simulink model "os_polyphase_synth_4096c"  (R2021a) (continued)
== system os_polyphase_synth_4096c/pfb_fir/pol1_in1_tap13 == (continued)
      | P>>17-(ACIN+D)*BCIN-C-CARRYIN
      | P>>17-(ACIN+D)*BCIN-CARRYIN
      | P>>17-(ACIN+D)*BCIN-P
      | P>>17-(ACIN+D)*BCIN-P-CARRYIN
      | P>>17-(ACIN+D)-C
      | P>>17-(ACIN+D)-C-CARRYIN
      | P>>17-(ACIN+D)-CARRYIN
      | P>>17-(ACIN+D)-P
      | P>>17-(ACIN+D)-P-CARRYIN
      | P>>17-(ACIN+P)
      | P>>17-(ACIN+P+CARRYIN)
      | P>>17-(B*(A+D))
      | P>>17-(B*(A+D)+C)
      | P>>17-(B*(A+D)+C+CARRYIN)
      | P>>17-(B*(A+D)+CARRYIN)
      | P>>17-(B*(A+D)+P)
      | P>>17-(B*(A+D)+P+CARRYIN)
      | P>>17-(B*(ACIN+D))
      | P>>17-(B*(ACIN+D)+C)
      | P>>17-(B*(ACIN+D)+C+CARRYIN)
      | P>>17-(B*(ACIN+D)+CARRYIN)
      | P>>17-(B*(ACIN+D)+P)
      | P>>17-(B*(ACIN+D)+P+CARRYIN)
      | P>>17-(B*(D+A))
      | P>>17-(B*(D+A)+C)
      | P>>17-(B*(D+A)+C+CARRYIN)
      | P>>17-(B*(D+A)+CARRYIN)
      | P>>17-(B*(D+A)+P)
      | P>>17-(B*(D+A)+P+CARRYIN)
      | P>>17-(B*(D+ACIN))
      | P>>17-(B*(D+ACIN)+C)
      | P>>17-(B*(D+ACIN)+C+CARRYIN)
      | P>>17-(B*(D+ACIN)+CARRYIN)
      | P>>17-(B*(D+ACIN)+P)
      | P>>17-(B*(D+ACIN)+P+CARRYIN)
      | P>>17-(B*(D-A))
      | P>>17-(B*(D-A)+C)
      | P>>17-(B*(D-A)+C+CARRYIN)
      | P>>17-(B*(D-A)+CARRYIN)
      | P>>17-(B*(D-A)+P)
      | P>>17-(B*(D-A)+P+CARRYIN)
      | P>>17-(B*(D-ACIN))
      | P>>17-(B*(D-ACIN)+C)
      | P>>17-(B*(D-ACIN)+C+CARRYIN)
      | P>>17-(B*(D-ACIN)+CARRYIN)
      | P>>17-(B*(D-ACIN)+P)
      | P>>17-(B*(D-ACIN)+P+CARRYIN)
      | P>>17-(B*A)
      | P>>17-(B*A+C)
      | P>>17-(B*A+C+CARRYIN)
      | P>>17-(B*A+CARRYIN)
      | P>>17-(B*A+P)
      | P>>17-(B*A+P+CARRYIN)
      | P>>17-(B*ACIN)
      | P>>17-(B*ACIN+C)
      | P>>17-(B*ACIN+C+CARRYIN)
      | P>>17-(B*ACIN+CARRYIN)
      | P>>17-(B*ACIN+P)
      | P>>17-(B*ACIN+P+CARRYIN)
      | P>>17-(B*D)
      | P>>17-(B*D+C)
      | P>>17-(B*D+C+CARRYIN)
      | P>>17-(B*D+CARRYIN)
      | P>>17-(B*D+P)
      | P>>17-(B*D+P+CARRYIN)
      | P>>17-(BCIN*(A+D))
      | P>>17-(BCIN*(A+D)+C)
      | P>>17-(BCIN*(A+D)+C+CARRYIN)
      | P>>17-(BCIN*(A+D)+CARRYIN)
      | P>>17-(BCIN*(A+D)+P)
      | P>>17-(BCIN*(A+D)+P+CARRYIN)
      | P>>17-(BCIN*(ACIN+D))
      | P>>17-(BCIN*(ACIN+D)+C)
      | P>>17-(BCIN*(ACIN+D)+C+CARRYIN)
      | P>>17-(BCIN*(ACIN+D)+CARRYIN)
      | P>>17-(BCIN*(ACIN+D)+P)
      | P>>17-(BCIN*(ACIN+D)+P+CARRYIN)
      | P>>17-(BCIN*(D+A))
      | P>>17-(BCIN*(D+A)+C)
      | P>>17-(BCIN*(D+A)+C+CARRYIN)
      | P>>17-(BCIN*(D+A)+CARRYIN)
      | P>>17-(BCIN*(D+A)+P)
      | P>>17-(BCIN*(D+A)+P+CARRYIN)
      | P>>17-(BCIN*(D+ACIN))
      | P>>17-(BCIN*(D+ACIN)+C)
      | P>>17-(BCIN*(D+ACIN)+C+CARRYIN)
      | P>>17-(BCIN*(D+ACIN)+CARRYIN)
      | P>>17-(BCIN*(D+ACIN)+P)
      | P>>17-(BCIN*(D+ACIN)+P+CARRYIN)
      | P>>17-(BCIN*(D-A))
      | P>>17-(BCIN*(D-A)+C)
      | P>>17-(BCIN*(D-A)+C+CARRYIN)
      | P>>17-(BCIN*(D-A)+CARRYIN)
      | P>>17-(BCIN*(D-A)+P)
      | P>>17-(BCIN*(D-A)+P+CARRYIN)
      | P>>17-(BCIN*(D-ACIN))
      | P>>17-(BCIN*(D-ACIN)+C)
      | P>>17-(BCIN*(D-ACIN)+C+CARRYIN)
      | P>>17-(BCIN*(D-ACIN)+CARRYIN)
      | P>>17-(BCIN*(D-ACIN)+P)
      | P>>17-(BCIN*(D-ACIN)+P+CARRYIN)
      | P>>17-(BCIN*A)
      | P>>17-(BCIN*A+C)
      | P>>17-(BCIN*A+C+CARRYIN)
      | P>>17-(BCIN*A+CARRYIN)
      | P>>17-(BCIN*A+P)
      | P>>17-(BCIN*A+P+CARRYIN)
      | P>>17-(BCIN*ACIN)
      | P>>17-(BCIN*ACIN+C)
      | P>>17-(BCIN*ACIN+C+CARRYIN)
      | P>>17-(BCIN*ACIN+CARRYIN)
      | P>>17-(BCIN*ACIN+P)
      | P>>17-(BCIN*ACIN+P+CARRYIN)
      | P>>17-(BCIN*D)
      | P>>17-(BCIN*D+C)
      | P>>17-(BCIN*D+C+CARRYIN)
      | P>>17-(BCIN*D+CARRYIN)
      | P>>17-(BCIN*D+P)
      | P>>17-(BCIN*D+P+CARRYIN)
      | P>>17-(C)
      | P>>17-(C+(A+D))
      | P>>17-(C+(A+D)*(A+D))
      | P>>17-(C+(A+D)*(A+D)+CARRYIN)
      | P>>17-(C+(A+D)*B)
      | P>>17-(C+(A+D)*B+CARRYIN)
      | P>>17-(C+(A+D)*BCIN)
      | P>>17-(C+(A+D)*BCIN+CARRYIN)
      | P>>17-(C+(A+D)+CARRYIN)
      | P>>17-(C+(ACIN+D))
      | P>>17-(C+(ACIN+D)*(ACIN+D))
      | P>>17-(C+(ACIN+D)*(ACIN+D)+CARRYIN)
      | P>>17-(C+(ACIN+D)*B)
      | P>>17-(C+(ACIN+D)*B+CARRYIN)
      | P>>17-(C+(ACIN+D)*BCIN)
      | P>>17-(C+(ACIN+D)*BCIN+CARRYIN)
      | P>>17-(C+(ACIN+D)+CARRYIN)
      | P>>17-(C+(D+A))
      | P>>17-(C+(D+A)*(D+A))
      | P>>17-(C+(D+A)*(D+A)+CARRYIN)
      | P>>17-(C+(D+A)*B)
      | P>>17-(C+(D+A)*B+CARRYIN)
      | P>>17-(C+(D+A)*BCIN)
      | P>>17-(C+(D+A)*BCIN+CARRYIN)
      | P>>17-(C+(D+A)+CARRYIN)
      | P>>17-(C+(D+ACIN))
      | P>>17-(C+(D+ACIN)*(D+ACIN))
      | P>>17-(C+(D+ACIN)*(D+ACIN)+CARRYIN)
      | P>>17-(C+(D+ACIN)*B)
      | P>>17-(C+(D+ACIN)*B+CARRYIN)
      | P>>17-(C+(D+ACIN)*BCIN)
      | P>>17-(C+(D+ACIN)*BCIN+CARRYIN)
      | P>>17-(C+(D+ACIN)+CARRYIN)
      | P>>17-(C+(D-A))
      | P>>17-(C+(D-A)*(D-A))
      | P>>17-(C+(D-A)*(D-A)+CARRYIN)
      | P>>17-(C+(D-A)*B)
      | P>>17-(C+(D-A)*B+CARRYIN)
      | P>>17-(C+(D-A)*BCIN)
      | P>>17-(C+(D-A)*BCIN+CARRYIN)
      | P>>17-(C+(D-A)+CARRYIN)
      | P>>17-(C+(D-ACIN))
      | P>>17-(C+(D-ACIN)*(D-ACIN))
      | P>>17-(C+(D-ACIN)*(D-ACIN)+CARRYIN)
      | P>>17-(C+(D-ACIN)*B)
      | P>>17-(C+(D-ACIN)*B+CARRYIN)
      | P>>17-(C+(D-ACIN)*BCIN)
      | P>>17-(C+(D-ACIN)*BCIN+CARRYIN)
      | P>>17-(C+(D-ACIN)+CARRYIN)
      | P>>17-(C+A)
      | P>>17-(C+A*A)
      | P>>17-(C+A*A+CARRYIN)
      | P>>17-(C+A*B)
      | P>>17-(C+A*B+CARRYIN)
      | P>>17-(C+A*BCIN)
      | P>>17-(C+A*BCIN+CARRYIN)
      | P>>17-(C+A+CARRYIN)
      | P>>17-(C+ACIN)
      | P>>17-(C+ACIN*ACIN)
      | P>>17-(C+ACIN*ACIN+CARRYIN)
      | P>>17-(C+ACIN*B)
      | P>>17-(C+ACIN*B+CARRYIN)
      | P>>17-(C+ACIN*BCIN)
      | P>>17-(C+ACIN*BCIN+CARRYIN)
      | P>>17-(C+ACIN+CARRYIN)
      | P>>17-(C+B*(A+D))
      | P>>17-(C+B*(A+D)+CARRYIN)
      | P>>17-(C+B*(ACIN+D))
      | P>>17-(C+B*(ACIN+D)+CARRYIN)
      | P>>17-(C+B*(D+A))
      | P>>17-(C+B*(D+A)+CARRYIN)
      | P>>17-(C+B*(D+ACIN))
      | P>>17-(C+B*(D+ACIN)+CARRYIN)
      | P>>17-(C+B*(D-A))
      | P>>17-(C+B*(D-A)+CARRYIN)
      | P>>17-(C+B*(D-ACIN))
      | P>>17-(C+B*(D-ACIN)+CARRYIN)
      | P>>17-(C+B*A)
      | P>>17-(C+B*A+CARRYIN)
      | P>>17-(C+B*ACIN)
      | P>>17-(C+B*ACIN+CARRYIN)
      | P>>17-(C+B*D)
      | P>>17-(C+B*D+CARRYIN)
      | P>>17-(C+BCIN*(A+D))
      | P>>17-(C+BCIN*(A+D)+CARRYIN)
      | P>>17-(C+BCIN*(ACIN+D))
      | P>>17-(C+BCIN*(ACIN+D)+CARRYIN)
      | P>>17-(C+BCIN*(D+A))
      | P>>17-(C+BCIN*(D+A)+CARRYIN)
      | P>>17-(C+BCIN*(D+ACIN))
      | P>>17-(C+BCIN*(D+ACIN)+CARRYIN)
      | P>>17-(C+BCIN*(D-A))
      | P>>17-(C+BCIN*(D-A)+CARRYIN)
      | P>>17-(C+BCIN*(D-ACIN))
      | P>>17-(C+BCIN*(D-ACIN)+CARRYIN)
      | P>>17-(C+BCIN*A)
      | P>>17-(C+BCIN*A+CARRYIN)
      | P>>17-(C+BCIN*ACIN)
      | P>>17-(C+BCIN*ACIN+CARRYIN)
      | P>>17-(C+BCIN*D)
      | P>>17-(C+BCIN*D+CARRYIN)
      | P>>17-(C+C)
      | P>>17-(C+C+CARRYIN)
      | P>>17-(C+C+CONCAT)
      | P>>17-(C+C+CONCAT+CARRYIN)
      | P>>17-(C+C+P)
      | P>>17-(C+C+P+CARRYIN)
      | P>>17-(C+CARRYIN)
      | P>>17-(C+CONCAT)
      | P>>17-(C+CONCAT+C)
      | P>>17-(C+CONCAT+C+CARRYIN)
      | P>>17-(C+CONCAT+CARRYIN)
      | P>>17-(C+CONCAT+P)
      | P>>17-(C+CONCAT+P+CARRYIN)
      | P>>17-(C+D)
      | P>>17-(C+D*B)
      | P>>17-(C+D*B+CARRYIN)
      | P>>17-(C+D*BCIN)
      | P>>17-(C+D*BCIN+CARRYIN)
      | P>>17-(C+D*D)
      | P>>17-(C+D*D+CARRYIN)
      | P>>17-(C+D+CARRYIN)
      | P>>17-(C+P)
      | P>>17-(C+P+C)
      | P>>17-(C+P+C+CARRYIN)
      | P>>17-(C+P+CARRYIN)
      | P>>17-(C+P+CONCAT)
      | P>>17-(C+P+CONCAT+CARRYIN)
      | P>>17-(C+P+P)
      | P>>17-(C+P+P+CARRYIN)
      | P>>17-(CARRYIN)
      | P>>17-(CONCAT)
      | P>>17-(CONCAT+C)
      | P>>17-(CONCAT+C+C)
      | P>>17-(CONCAT+C+C+CARRYIN)
      | P>>17-(CONCAT+C+CARRYIN)
      | P>>17-(CONCAT+C+P)
      | P>>17-(CONCAT+C+P+CARRYIN)
      | P>>17-(CONCAT+CARRYIN)
      | P>>17-(CONCAT+P)
      | P>>17-(CONCAT+P+C)
      | P>>17-(CONCAT+P+C+CARRYIN)
      | P>>17-(CONCAT+P+CARRYIN)
      | P>>17-(D)
      | P>>17-(D*B)
      | P>>17-(D*B+C)
      | P>>17-(D*B+C+CARRYIN)
      | P>>17-(D*B+CARRYIN)
      | P>>17-(D*B+P)
      | P>>17-(D*B+P+CARRYIN)
      | P>>17-(D*BCIN)
      | P>>17-(D*BCIN+C)
      | P>>17-(D*BCIN+C+CARRYIN)
      | P>>17-(D*BCIN+CARRYIN)
      | P>>17-(D*BCIN+P)
      | P>>17-(D*BCIN+P+CARRYIN)
      | P>>17-(D*D)
      | P>>17-(D*D+C)
      | P>>17-(D*D+C+CARRYIN)
      | P>>17-(D*D+CARRYIN)
      | P>>17-(D*D+P)
      | P>>17-(D*D+P+CARRYIN)
      | P>>17-(D+A)
      | P>>17-(D+A)*(D+A)
      | P>>17-(D+A)*(D+A)-C
      | P>>17-(D+A)*(D+A)-C-CARRYIN
      | P>>17-(D+A)*(D+A)-CARRYIN
      | P>>17-(D+A)*(D+A)-P
      | P>>17-(D+A)*(D+A)-P-CARRYIN
      | P>>17-(D+A)*B
      | P>>17-(D+A)*B-C
      | P>>17-(D+A)*B-C-CARRYIN
      | P>>17-(D+A)*B-CARRYIN
      | P>>17-(D+A)*B-P
      | P>>17-(D+A)*B-P-CARRYIN
      | P>>17-(D+A)*BCIN
      | P>>17-(D+A)*BCIN-C
      | P>>17-(D+A)*BCIN-C-CARRYIN
      | P>>17-(D+A)*BCIN-CARRYIN
      | P>>17-(D+A)*BCIN-P
      | P>>17-(D+A)*BCIN-P-CARRYIN
      | P>>17-(D+A)-C
      | P>>17-(D+A)-C-CARRYIN
      | P>>17-(D+A)-CARRYIN
      | P>>17-(D+A)-P
      | P>>17-(D+A)-P-CARRYIN
      | P>>17-(D+ACIN)
      | P>>17-(D+ACIN)*(D+ACIN)
      | P>>17-(D+ACIN)*(D+ACIN)-C
      | P>>17-(D+ACIN)*(D+ACIN)-C-CARRYIN
      | P>>17-(D+ACIN)*(D+ACIN)-CARRYIN
      | P>>17-(D+ACIN)*(D+ACIN)-P
      | P>>17-(D+ACIN)*(D+ACIN)-P-CARRYIN
      | P>>17-(D+ACIN)*B
      | P>>17-(D+ACIN)*B-C
      | P>>17-(D+ACIN)*B-C-CARRYIN
      | P>>17-(D+ACIN)*B-CARRYIN
      | P>>17-(D+ACIN)*B-P
      | P>>17-(D+ACIN)*B-P-CARRYIN
      | P>>17-(D+ACIN)*BCIN
      | P>>17-(D+ACIN)*BCIN-C
      | P>>17-(D+ACIN)*BCIN-C-CARRYIN
      | P>>17-(D+ACIN)*BCIN-CARRYIN
      | P>>17-(D+ACIN)*BCIN-P
      | P>>17-(D+ACIN)*BCIN-P-CARRYIN
      | P>>17-(D+ACIN)-C
      | P>>17-(D+ACIN)-C-CARRYIN
      | P>>17-(D+ACIN)-CARRYIN
      | P>>17-(D+ACIN)-P
      | P>>17-(D+ACIN)-P-CARRYIN
      | P>>17-(D+C)
      | P>>17-(D+C+CARRYIN)
      | P>>17-(D+CARRYIN)
      | P>>17-(D+P)
      | P>>17-(D+P+CARRYIN)
      | P>>17-(D-A)
      | P>>17-(D-A)*(D-A)
      | P>>17-(D-A)*(D-A)-C
      | P>>17-(D-A)*(D-A)-C-CARRYIN
      | P>>17-(D-A)*(D-A)-CARRYIN
      | P>>17-(D-A)*(D-A)-P
      | P>>17-(D-A)*(D-A)-P-CARRYIN
      | P>>17-(D-A)*B
      | P>>17-(D-A)*B-C
      | P>>17-(D-A)*B-C-CARRYIN
      | P>>17-(D-A)*B-CARRYIN
      | P>>17-(D-A)*B-P
      | P>>17-(D-A)*B-P-CARRYIN
      | P>>17-(D-A)*BCIN
      | P>>17-(D-A)*BCIN-C
      | P>>17-(D-A)*BCIN-C-CARRYIN
      | P>>17-(D-A)*BCIN-CARRYIN
      | P>>17-(D-A)*BCIN-P
      | P>>17-(D-A)*BCIN-P-CARRYIN
      | P>>17-(D-A)-C
      | P>>17-(D-A)-C-CARRYIN
      | P>>17-(D-A)-CARRYIN
      | P>>17-(D-A)-P
      | P>>17-(D-A)-P-CARRYIN
      | P>>17-(D-ACIN)
      | P>>17-(D-ACIN)*(D-ACIN)
      | P>>17-(D-ACIN)*(D-ACIN)-C
      | P>>17-(D-ACIN)*(D-ACIN)-C-CARRYIN
      | P>>17-(D-ACIN)*(D-ACIN)-CARRYIN
      | P>>17-(D-ACIN)*(D-ACIN)-P
      | P>>17-(D-ACIN)*(D-ACIN)-P-CARRYIN
      | P>>17-(D-ACIN)*B
      | P>>17-(D-ACIN)*B-C
      | P>>17-(D-ACIN)*B-C-CARRYIN
      | P>>17-(D-ACIN)*B-CARRYIN
      | P>>17-(D-ACIN)*B-P
      | P>>17-(D-ACIN)*B-P-CARRYIN
      | P>>17-(D-ACIN)*BCIN
      | P>>17-(D-ACIN)*BCIN-C
      | P>>17-(D-ACIN)*BCIN-C-CARRYIN
      | P>>17-(D-ACIN)*BCIN-CARRYIN
      | P>>17-(D-ACIN)*BCIN-P
      | P>>17-(D-ACIN)*BCIN-P-CARRYIN
      | P>>17-(D-ACIN)-C
      | P>>17-(D-ACIN)-C-CARRYIN
      | P>>17-(D-ACIN)-CARRYIN
      | P>>17-(D-ACIN)-P
      | P>>17-(D-ACIN)-P-CARRYIN
      | P>>17-(P)
      | P>>17-(P+(A+D))
      | P>>17-(P+(A+D)*(A+D))
      | P>>17-(P+(A+D)*(A+D)+CARRYIN)
      | P>>17-(P+(A+D)*B)
      | P>>17-(P+(A+D)*B+CARRYIN)
      | P>>17-(P+(A+D)*BCIN)
      | P>>17-(P+(A+D)*BCIN+CARRYIN)
      | P>>17-(P+(A+D)+CARRYIN)
      | P>>17-(P+(ACIN+D))
      | P>>17-(P+(ACIN+D)*(ACIN+D))
      | P>>17-(P+(ACIN+D)*(ACIN+D)+CARRYIN)
      | P>>17-(P+(ACIN+D)*B)
      | P>>17-(P+(ACIN+D)*B+CARRYIN)
      | P>>17-(P+(ACIN+D)*BCIN)
      | P>>17-(P+(ACIN+D)*BCIN+CARRYIN)
      | P>>17-(P+(ACIN+D)+CARRYIN)
      | P>>17-(P+(D+A))
      | P>>17-(P+(D+A)*(D+A))
      | P>>17-(P+(D+A)*(D+A)+CARRYIN)
      | P>>17-(P+(D+A)*B)
      | P>>17-(P+(D+A)*B+CARRYIN)
      | P>>17-(P+(D+A)*BCIN)
      | P>>17-(P+(D+A)*BCIN+CARRYIN)
      | P>>17-(P+(D+A)+CARRYIN)
      | P>>17-(P+(D+ACIN))
      | P>>17-(P+(D+ACIN)*(D+ACIN))
      | P>>17-(P+(D+ACIN)*(D+ACIN)+CARRYIN)
      | P>>17-(P+(D+ACIN)*B)
      | P>>17-(P+(D+ACIN)*B+CARRYIN)
      | P>>17-(P+(D+ACIN)*BCIN)
      | P>>17-(P+(D+ACIN)*BCIN+CARRYIN)
      | P>>17-(P+(D+ACIN)+CARRYIN)
      | P>>17-(P+(D-A))
      | P>>17-(P+(D-A)*(D-A))
      | P>>17-(P+(D-A)*(D-A)+CARRYIN)
      | P>>17-(P+(D-A)*B)
      | P>>17-(P+(D-A)*B+CARRYIN)
      | P>>17-(P+(D-A)*BCIN)
      | P>>17-(P+(D-A)*BCIN+CARRYIN)
      | P>>17-(P+(D-A)+CARRYIN)
      | P>>17-(P+(D-ACIN))
      | P>>17-(P+(D-ACIN)*(D-ACIN))
      | P>>17-(P+(D-ACIN)*(D-ACIN)+CARRYIN)
      | P>>17-(P+(D-ACIN)*B)
      | P>>17-(P+(D-ACIN)*B+CARRYIN)
      | P>>17-(P+(D-ACIN)*BCIN)
      | P>>17-(P+(D-ACIN)*BCIN+CARRYIN)
      | P>>17-(P+(D-ACIN)+CARRYIN)
      | P>>17-(P+A)
      | P>>17-(P+A*A)
      | P>>17-(P+A*A+CARRYIN)
      | P>>17-(P+A*B)
      | P>>17-(P+A*B+CARRYIN)
      | P>>17-(P+A*BCIN)
      | P>>17-(P+A*BCIN+CARRYIN)
      | P>>17-(P+A+CARRYIN)
      | P>>17-(P+ACIN)
      | P>>17-(P+ACIN*ACIN)
      | P>>17-(P+ACIN*ACIN+CARRYIN)
      | P>>17-(P+ACIN*B)
      | P>>17-(P+ACIN*B+CARRYIN)
      | P>>17-(P+ACIN*BCIN)
      | P>>17-(P+ACIN*BCIN+CARRYIN)
      | P>>17-(P+ACIN+CARRYIN)
      | P>>17-(P+B*(A+D))
      | P>>17-(P+B*(A+D)+CARRYIN)
      | P>>17-(P+B*(ACIN+D))
      | P>>17-(P+B*(ACIN+D)+CARRYIN)
      | P>>17-(P+B*(D+A))
      | P>>17-(P+B*(D+A)+CARRYIN)
      | P>>17-(P+B*(D+ACIN))
      | P>>17-(P+B*(D+ACIN)+CARRYIN)
      | P>>17-(P+B*(D-A))
      | P>>17-(P+B*(D-A)+CARRYIN)
      | P>>17-(P+B*(D-ACIN))
      | P>>17-(P+B*(D-ACIN)+CARRYIN)
      | P>>17-(P+B*A)
      | P>>17-(P+B*A+CARRYIN)
      | P>>17-(P+B*ACIN)
      | P>>17-(P+B*ACIN+CARRYIN)
      | P>>17-(P+B*D)
      | P>>17-(P+B*D+CARRYIN)
      | P>>17-(P+BCIN*(A+D))
      | P>>17-(P+BCIN*(A+D)+CARRYIN)
      | P>>17-(P+BCIN*(ACIN+D))
      | P>>17-(P+BCIN*(ACIN+D)+CARRYIN)
      | P>>17-(P+BCIN*(D+A))
      | P>>17-(P+BCIN*(D+A)+CARRYIN)
      | P>>17-(P+BCIN*(D+ACIN))
      | P>>17-(P+BCIN*(D+ACIN)+CARRYIN)
      | P>>17-(P+BCIN*(D-A))
      | P>>17-(P+BCIN*(D-A)+CARRYIN)
      | P>>17-(P+BCIN*(D-ACIN))
      | P>>17-(P+BCIN*(D-ACIN)+CARRYIN)
      | P>>17-(P+BCIN*A)
      | P>>17-(P+BCIN*A+CARRYIN)
      | P>>17-(P+BCIN*ACIN)
      | P>>17-(P+BCIN*ACIN+CARRYIN)
      | P>>17-(P+BCIN*D)
      | P>>17-(P+BCIN*D+CARRYIN)
      | P>>17-(P+C)
      | P>>17-(P+C+C)
      | P>>17-(P+C+C+CARRYIN)
      | P>>17-(P+C+CARRYIN)
      | P>>17-(P+C+CONCAT)
      | P>>17-(P+C+CONCAT+CARRYIN)
      | P>>17-(P+C+P)
      | P>>17-(P+C+P+CARRYIN)
      | P>>17-(P+CARRYIN)
      | P>>17-(P+CONCAT)
      | P>>17-(P+CONCAT+C)
      | P>>17-(P+CONCAT+C+CARRYIN)
      | P>>17-(P+CONCAT+CARRYIN)
      | P>>17-(P+D)
      | P>>17-(P+D*B)
      | P>>17-(P+D*B+CARRYIN)
      | P>>17-(P+D*BCIN)
      | P>>17-(P+D*BCIN+CARRYIN)
      | P>>17-(P+D*D)
      | P>>17-(P+D*D+CARRYIN)
      | P>>17-(P+D+CARRYIN)
      | P>>17-(P+P)
      | P>>17-(P+P+C)
      | P>>17-(P+P+C+CARRYIN)
      | P>>17-(P+P+CARRYIN)
      | P>>17-A
      | P>>17-A*A
      | P>>17-A*A-C
      | P>>17-A*A-C-CARRYIN
      | P>>17-A*A-CARRYIN
      | P>>17-A*A-P
      | P>>17-A*A-P-CARRYIN
      | P>>17-A*B
      | P>>17-A*B-C
      | P>>17-A*B-C-CARRYIN
      | P>>17-A*B-CARRYIN
      | P>>17-A*B-P
      | P>>17-A*B-P-CARRYIN
      | P>>17-A*BCIN
      | P>>17-A*BCIN-C
      | P>>17-A*BCIN-C-CARRYIN
      | P>>17-A*BCIN-CARRYIN
      | P>>17-A*BCIN-P
      | P>>17-A*BCIN-P-CARRYIN
      | P>>17-A-C
      | P>>17-A-C-CARRYIN
      | P>>17-A-CARRYIN
      | P>>17-A-P
      | P>>17-A-P-CARRYIN
      | P>>17-ACIN
      | P>>17-ACIN*ACIN
      | P>>17-ACIN*ACIN-C
      | P>>17-ACIN*ACIN-C-CARRYIN
      | P>>17-ACIN*ACIN-CARRYIN
      | P>>17-ACIN*ACIN-P
      | P>>17-ACIN*ACIN-P-CARRYIN
      | P>>17-ACIN*B
      | P>>17-ACIN*B-C
      | P>>17-ACIN*B-C-CARRYIN
      | P>>17-ACIN*B-CARRYIN
      | P>>17-ACIN*B-P
      | P>>17-ACIN*B-P-CARRYIN
      | P>>17-ACIN*BCIN
      | P>>17-ACIN*BCIN-C
      | P>>17-ACIN*BCIN-C-CARRYIN
      | P>>17-ACIN*BCIN-CARRYIN
      | P>>17-ACIN*BCIN-P
      | P>>17-ACIN*BCIN-P-CARRYIN
      | P>>17-ACIN-C
      | P>>17-ACIN-C-CARRYIN
      | P>>17-ACIN-CARRYIN
      | P>>17-ACIN-P
      | P>>17-ACIN-P-CARRYIN
      | P>>17-B*(A+D)
      | P>>17-B*(A+D)-C
      | P>>17-B*(A+D)-C-CARRYIN
      | P>>17-B*(A+D)-CARRYIN
      | P>>17-B*(A+D)-P
      | P>>17-B*(A+D)-P-CARRYIN
      | P>>17-B*(ACIN+D)
      | P>>17-B*(ACIN+D)-C
      | P>>17-B*(ACIN+D)-C-CARRYIN
      | P>>17-B*(ACIN+D)-CARRYIN
      | P>>17-B*(ACIN+D)-P
      | P>>17-B*(ACIN+D)-P-CARRYIN
      | P>>17-B*(D+A)
      | P>>17-B*(D+A)-C
      | P>>17-B*(D+A)-C-CARRYIN
      | P>>17-B*(D+A)-CARRYIN
      | P>>17-B*(D+A)-P
      | P>>17-B*(D+A)-P-CARRYIN
      | P>>17-B*(D+ACIN)
      | P>>17-B*(D+ACIN)-C
      | P>>17-B*(D+ACIN)-C-CARRYIN
      | P>>17-B*(D+ACIN)-CARRYIN
      | P>>17-B*(D+ACIN)-P
      | P>>17-B*(D+ACIN)-P-CARRYIN
      | P>>17-B*(D-A)
      | P>>17-B*(D-A)-C
      | P>>17-B*(D-A)-C-CARRYIN
      | P>>17-B*(D-A)-CARRYIN
      | P>>17-B*(D-A)-P
      | P>>17-B*(D-A)-P-CARRYIN
      | P>>17-B*(D-ACIN)
      | P>>17-B*(D-ACIN)-C
      | P>>17-B*(D-ACIN)-C-CARRYIN
      | P>>17-B*(D-ACIN)-CARRYIN
      | P>>17-B*(D-ACIN)-P
      | P>>17-B*(D-ACIN)-P-CARRYIN
      | P>>17-B*A
      | P>>17-B*A-C
      | P>>17-B*A-C-CARRYIN
      | P>>17-B*A-CARRYIN
      | P>>17-B*A-P
      | P>>17-B*A-P-CARRYIN
      | P>>17-B*ACIN
      | P>>17-B*ACIN-C
      | P>>17-B*ACIN-C-CARRYIN
      | P>>17-B*ACIN-CARRYIN
      | P>>17-B*ACIN-P
      | P>>17-B*ACIN-P-CARRYIN
      | P>>17-B*D
      | P>>17-B*D-C
      | P>>17-B*D-C-CARRYIN
      | P>>17-B*D-CARRYIN
      | P>>17-B*D-P
      | P>>17-B*D-P-CARRYIN
      | P>>17-BCIN*(A+D)
      | P>>17-BCIN*(A+D)-C
      | P>>17-BCIN*(A+D)-C-CARRYIN
      | P>>17-BCIN*(A+D)-CARRYIN
      | P>>17-BCIN*(A+D)-P
      | P>>17-BCIN*(A+D)-P-CARRYIN
      | P>>17-BCIN*(ACIN+D)
      | P>>17-BCIN*(ACIN+D)-C
      | P>>17-BCIN*(ACIN+D)-C-CARRYIN
      | P>>17-BCIN*(ACIN+D)-CARRYIN
      | P>>17-BCIN*(ACIN+D)-P
      | P>>17-BCIN*(ACIN+D)-P-CARRYIN
      | P>>17-BCIN*(D+A)
      | P>>17-BCIN*(D+A)-C
      | P>>17-BCIN*(D+A)-C-CARRYIN
      | P>>17-BCIN*(D+A)-CARRYIN
      | P>>17-BCIN*(D+A)-P
      | P>>17-BCIN*(D+A)-P-CARRYIN
      | P>>17-BCIN*(D+ACIN)
      | P>>17-BCIN*(D+ACIN)-C
      | P>>17-BCIN*(D+ACIN)-C-CARRYIN
      | P>>17-BCIN*(D+ACIN)-CARRYIN
      | P>>17-BCIN*(D+ACIN)-P
      | P>>17-BCIN*(D+ACIN)-P-CARRYIN
      | P>>17-BCIN*(D-A)
      | P>>17-BCIN*(D-A)-C
      | P>>17-BCIN*(D-A)-C-CARRYIN
      | P>>17-BCIN*(D-A)-CARRYIN
      | P>>17-BCIN*(D-A)-P
      | P>>17-BCIN*(D-A)-P-CARRYIN
      | P>>17-BCIN*(D-ACIN)
      | P>>17-BCIN*(D-ACIN)-C
      | P>>17-BCIN*(D-ACIN)-C-CARRYIN
      | P>>17-BCIN*(D-ACIN)-CARRYIN
      | P>>17-BCIN*(D-ACIN)-P
      | P>>17-BCIN*(D-ACIN)-P-CARRYIN
      | P>>17-BCIN*A
      | P>>17-BCIN*A-C
      | P>>17-BCIN*A-C-CARRYIN
      | P>>17-BCIN*A-CARRYIN
      | P>>17-BCIN*A-P
      | P>>17-BCIN*A-P-CARRYIN
      | P>>17-BCIN*ACIN
      | P>>17-BCIN*ACIN-C
      | P>>17-BCIN*ACIN-C-CARRYIN
      | P>>17-BCIN*ACIN-CARRYIN
      | P>>17-BCIN*ACIN-P
      | P>>17-BCIN*ACIN-P-CARRYIN
      | P>>17-BCIN*D
      | P>>17-BCIN*D-C
      | P>>17-BCIN*D-C-CARRYIN
      | P>>17-BCIN*D-CARRYIN
      | P>>17-BCIN*D-P
      | P>>17-BCIN*D-P-CARRYIN
      | P>>17-C
      | P>>17-C-(A+D)
      | P>>17-C-(A+D)*(A+D)
      | P>>17-C-(A+D)*(A+D)-CARRYIN
      | P>>17-C-(A+D)*B
      | P>>17-C-(A+D)*B-CARRYIN
      | P>>17-C-(A+D)*BCIN
      | P>>17-C-(A+D)*BCIN-CARRYIN
      | P>>17-C-(A+D)-CARRYIN
      | P>>17-C-(ACIN+D)
      | P>>17-C-(ACIN+D)*(ACIN+D)
      | P>>17-C-(ACIN+D)*(ACIN+D)-CARRYIN
      | P>>17-C-(ACIN+D)*B
      | P>>17-C-(ACIN+D)*B-CARRYIN
      | P>>17-C-(ACIN+D)*BCIN
      | P>>17-C-(ACIN+D)*BCIN-CARRYIN
      | P>>17-C-(ACIN+D)-CARRYIN
      | P>>17-C-(D+A)
      | P>>17-C-(D+A)*(D+A)
      | P>>17-C-(D+A)*(D+A)-CARRYIN
      | P>>17-C-(D+A)*B
      | P>>17-C-(D+A)*B-CARRYIN
      | P>>17-C-(D+A)*BCIN
      | P>>17-C-(D+A)*BCIN-CARRYIN
      | P>>17-C-(D+A)-CARRYIN
      | P>>17-C-(D+ACIN)
      | P>>17-C-(D+ACIN)*(D+ACIN)
      | P>>17-C-(D+ACIN)*(D+ACIN)-CARRYIN
      | P>>17-C-(D+ACIN)*B
      | P>>17-C-(D+ACIN)*B-CARRYIN
      | P>>17-C-(D+ACIN)*BCIN
      | P>>17-C-(D+ACIN)*BCIN-CARRYIN
      | P>>17-C-(D+ACIN)-CARRYIN
      | P>>17-C-(D-A)
      | P>>17-C-(D-A)*(D-A)
      | P>>17-C-(D-A)*(D-A)-CARRYIN
      | P>>17-C-(D-A)*B
      | P>>17-C-(D-A)*B-CARRYIN
      | P>>17-C-(D-A)*BCIN
      | P>>17-C-(D-A)*BCIN-CARRYIN
      | P>>17-C-(D-A)-CARRYIN
      | P>>17-C-(D-ACIN)
      | P>>17-C-(D-ACIN)*(D-ACIN)
      | P>>17-C-(D-ACIN)*(D-ACIN)-CARRYIN
      | P>>17-C-(D-ACIN)*B
      | P>>17-C-(D-ACIN)*B-CARRYIN
      | P>>17-C-(D-ACIN)*BCIN
      | P>>17-C-(D-ACIN)*BCIN-CARRYIN
      | P>>17-C-(D-ACIN)-CARRYIN
      | P>>17-C-A
      | P>>17-C-A*A
      | P>>17-C-A*A-CARRYIN
      | P>>17-C-A*B
      | P>>17-C-A*B-CARRYIN
      | P>>17-C-A*BCIN
      | P>>17-C-A*BCIN-CARRYIN
      | P>>17-C-A-CARRYIN
      | P>>17-C-ACIN
      | P>>17-C-ACIN*ACIN
      | P>>17-C-ACIN*ACIN-CARRYIN
      | P>>17-C-ACIN*B
      | P>>17-C-ACIN*B-CARRYIN
      | P>>17-C-ACIN*BCIN
      | P>>17-C-ACIN*BCIN-CARRYIN
      | P>>17-C-ACIN-CARRYIN
      | P>>17-C-B*(A+D)
      | P>>17-C-B*(A+D)-CARRYIN
      | P>>17-C-B*(ACIN+D)
      | P>>17-C-B*(ACIN+D)-CARRYIN
      | P>>17-C-B*(D+A)
      | P>>17-C-B*(D+A)-CARRYIN
      | P>>17-C-B*(D+ACIN)
      | P>>17-C-B*(D+ACIN)-CARRYIN
      | P>>17-C-B*(D-A)
      | P>>17-C-B*(D-A)-CARRYIN
      | P>>17-C-B*(D-ACIN)
      | P>>17-C-B*(D-ACIN)-CARRYIN
      | P>>17-C-B*A
      | P>>17-C-B*A-CARRYIN
      | P>>17-C-B*ACIN
      | P>>17-C-B*ACIN-CARRYIN
      | P>>17-C-B*D
      | P>>17-C-B*D-CARRYIN
      | P>>17-C-BCIN*(A+D)
      | P>>17-C-BCIN*(A+D)-CARRYIN
      | P>>17-C-BCIN*(ACIN+D)
      | P>>17-C-BCIN*(ACIN+D)-CARRYIN
      | P>>17-C-BCIN*(D+A)
      | P>>17-C-BCIN*(D+A)-CARRYIN
      | P>>17-C-BCIN*(D+ACIN)
      | P>>17-C-BCIN*(D+ACIN)-CARRYIN
      | P>>17-C-BCIN*(D-A)
      | P>>17-C-BCIN*(D-A)-CARRYIN
      | P>>17-C-BCIN*(D-ACIN)
      | P>>17-C-BCIN*(D-ACIN)-CARRYIN
      | P>>17-C-BCIN*A
      | P>>17-C-BCIN*A-CARRYIN
      | P>>17-C-BCIN*ACIN
      | P>>17-C-BCIN*ACIN-CARRYIN
      | P>>17-C-BCIN*D
      | P>>17-C-BCIN*D-CARRYIN
      | P>>17-C-C
      | P>>17-C-C-CARRYIN
      | P>>17-C-C-CONCAT
      | P>>17-C-C-CONCAT-CARRYIN
      | P>>17-C-C-P
      | P>>17-C-C-P-CARRYIN
      | P>>17-C-CARRYIN
      | P>>17-C-CONCAT
      | P>>17-C-CONCAT-C
      | P>>17-C-CONCAT-C-CARRYIN
      | P>>17-C-CONCAT-CARRYIN
      | P>>17-C-CONCAT-P
      | P>>17-C-CONCAT-P-CARRYIN
      | P>>17-C-D
      | P>>17-C-D*B
      | P>>17-C-D*B-CARRYIN
      | P>>17-C-D*BCIN
      | P>>17-C-D*BCIN-CARRYIN
      | P>>17-C-D*D
      | P>>17-C-D*D-CARRYIN
      | P>>17-C-D-CARRYIN
      | P>>17-C-P
      | P>>17-C-P-C
      | P>>17-C-P-C-CARRYIN
      | P>>17-C-P-CARRYIN
      | P>>17-C-P-CONCAT
      | P>>17-C-P-CONCAT-CARRYIN
      | P>>17-C-P-P
      | P>>17-C-P-P-CARRYIN
      | P>>17-CARRYIN
      | P>>17-CONCAT
      | P>>17-CONCAT-C
      | P>>17-CONCAT-C-C
      | P>>17-CONCAT-C-C-CARRYIN
      | P>>17-CONCAT-C-CARRYIN
      | P>>17-CONCAT-C-P
      | P>>17-CONCAT-C-P-CARRYIN
      | P>>17-CONCAT-CARRYIN
      | P>>17-CONCAT-P
      | P>>17-CONCAT-P-C
      | P>>17-CONCAT-P-C-CARRYIN
      | P>>17-CONCAT-P-CARRYIN
      | P>>17-D
      | P>>17-D*B
      | P>>17-D*B-C
      | P>>17-D*B-C-CARRYIN
      | P>>17-D*B-CARRYIN
      | P>>17-D*B-P
      | P>>17-D*B-P-CARRYIN
      | P>>17-D*BCIN
      | P>>17-D*BCIN-C
      | P>>17-D*BCIN-C-CARRYIN
      | P>>17-D*BCIN-CARRYIN
      | P>>17-D*BCIN-P
      | P>>17-D*BCIN-P-CARRYIN
      | P>>17-D*D
      | P>>17-D*D-C
      | P>>17-D*D-C-CARRYIN
      | P>>17-D*D-CARRYIN
      | P>>17-D*D-P
      | P>>17-D*D-P-CARRYIN
      | P>>17-D-C
      | P>>17-D-C-CARRYIN
      | P>>17-D-CARRYIN
      | P>>17-D-P
      | P>>17-D-P-CARRYIN
      | P>>17-P
      | P>>17-P-(A+D)
      | P>>17-P-(A+D)*(A+D)
      | P>>17-P-(A+D)*(A+D)-CARRYIN
      | P>>17-P-(A+D)*B
      | P>>17-P-(A+D)*B-CARRYIN
      | P>>17-P-(A+D)*BCIN
      | P>>17-P-(A+D)*BCIN-CARRYIN
      | P>>17-P-(A+D)-CARRYIN
      | P>>17-P-(ACIN+D)
      | P>>17-P-(ACIN+D)*(ACIN+D)
      | P>>17-P-(ACIN+D)*(ACIN+D)-CARRYIN
      | P>>17-P-(ACIN+D)*B
      | P>>17-P-(ACIN+D)*B-CARRYIN
      | P>>17-P-(ACIN+D)*BCIN
      | P>>17-P-(ACIN+D)*BCIN-CARRYIN
      | P>>17-P-(ACIN+D)-CARRYIN
      | P>>17-P-(D+A)
      | P>>17-P-(D+A)*(D+A)
      | P>>17-P-(D+A)*(D+A)-CARRYIN
      | P>>17-P-(D+A)*B
      | P>>17-P-(D+A)*B-CARRYIN
      | P>>17-P-(D+A)*BCIN
      | P>>17-P-(D+A)*BCIN-CARRYIN
      | P>>17-P-(D+A)-CARRYIN
      | P>>17-P-(D+ACIN)
      | P>>17-P-(D+ACIN)*(D+ACIN)
      | P>>17-P-(D+ACIN)*(D+ACIN)-CARRYIN
      | P>>17-P-(D+ACIN)*B
      | P>>17-P-(D+ACIN)*B-CARRYIN
      | P>>17-P-(D+ACIN)*BCIN
      | P>>17-P-(D+ACIN)*BCIN-CARRYIN
      | P>>17-P-(D+ACIN)-CARRYIN
      | P>>17-P-(D-A)
      | P>>17-P-(D-A)*(D-A)
      | P>>17-P-(D-A)*(D-A)-CARRYIN
      | P>>17-P-(D-A)*B
      | P>>17-P-(D-A)*B-CARRYIN
      | P>>17-P-(D-A)*BCIN
      | P>>17-P-(D-A)*BCIN-CARRYIN
      | P>>17-P-(D-A)-CARRYIN
      | P>>17-P-(D-ACIN)
      | P>>17-P-(D-ACIN)*(D-ACIN)
      | P>>17-P-(D-ACIN)*(D-ACIN)-CARRYIN
      | P>>17-P-(D-ACIN)*B
      | P>>17-P-(D-ACIN)*B-CARRYIN
      | P>>17-P-(D-ACIN)*BCIN
      | P>>17-P-(D-ACIN)*BCIN-CARRYIN
      | P>>17-P-(D-ACIN)-CARRYIN
      | P>>17-P-A
      | P>>17-P-A*A
      | P>>17-P-A*A-CARRYIN
      | P>>17-P-A*B
      | P>>17-P-A*B-CARRYIN
      | P>>17-P-A*BCIN
      | P>>17-P-A*BCIN-CARRYIN
      | P>>17-P-A-CARRYIN
      | P>>17-P-ACIN
      | P>>17-P-ACIN*ACIN
      | P>>17-P-ACIN*ACIN-CARRYIN
      | P>>17-P-ACIN*B
      | P>>17-P-ACIN*B-CARRYIN
      | P>>17-P-ACIN*BCIN
      | P>>17-P-ACIN*BCIN-CARRYIN
      | P>>17-P-ACIN-CARRYIN
      | P>>17-P-B*(A+D)
      | P>>17-P-B*(A+D)-CARRYIN
      | P>>17-P-B*(ACIN+D)
      | P>>17-P-B*(ACIN+D)-CARRYIN
      | P>>17-P-B*(D+A)
      | P>>17-P-B*(D+A)-CARRYIN
      | P>>17-P-B*(D+ACIN)
      | P>>17-P-B*(D+ACIN)-CARRYIN
      | P>>17-P-B*(D-A)
      | P>>17-P-B*(D-A)-CARRYIN
      | P>>17-P-B*(D-ACIN)
      | P>>17-P-B*(D-ACIN)-CARRYIN
      | P>>17-P-B*A
      | P>>17-P-B*A-CARRYIN
      | P>>17-P-B*ACIN
      | P>>17-P-B*ACIN-CARRYIN
      | P>>17-P-B*D
      | P>>17-P-B*D-CARRYIN
      | P>>17-P-BCIN*(A+D)
      | P>>17-P-BCIN*(A+D)-CARRYIN
      | P>>17-P-BCIN*(ACIN+D)
      | P>>17-P-BCIN*(ACIN+D)-CARRYIN
      | P>>17-P-BCIN*(D+A)
      | P>>17-P-BCIN*(D+A)-CARRYIN
      | P>>17-P-BCIN*(D+ACIN)
      | P>>17-P-BCIN*(D+ACIN)-CARRYIN
      | P>>17-P-BCIN*(D-A)
      | P>>17-P-BCIN*(D-A)-CARRYIN
      | P>>17-P-BCIN*(D-ACIN)
      | P>>17-P-BCIN*(D-ACIN)-CARRYIN
      | P>>17-P-BCIN*A
      | P>>17-P-BCIN*A-CARRYIN
      | P>>17-P-BCIN*ACIN
      | P>>17-P-BCIN*ACIN-CARRYIN
      | P>>17-P-BCIN*D
      | P>>17-P-BCIN*D-CARRYIN
      | P>>17-P-C
      | P>>17-P-C-C
      | P>>17-P-C-C-CARRYIN
      | P>>17-P-C-CARRYIN
      | P>>17-P-C-CONCAT
      | P>>17-P-C-CONCAT-CARRYIN
      | P>>17-P-C-P
      | P>>17-P-C-P-CARRYIN
      | P>>17-P-CARRYIN
      | P>>17-P-CONCAT
      | P>>17-P-CONCAT-C
      | P>>17-P-CONCAT-C-CARRYIN
      | P>>17-P-CONCAT-CARRYIN
      | P>>17-P-D
      | P>>17-P-D*B
      | P>>17-P-D*B-CARRYIN
      | P>>17-P-D*BCIN
      | P>>17-P-D*BCIN-CARRYIN
      | P>>17-P-D*D
      | P>>17-P-D*D-CARRYIN
      | P>>17-P-D-CARRYIN
      | P>>17-P-P
      | P>>17-P-P-C
      | P>>17-P-P-C-CARRYIN
      | P>>17-P-P-CARRYIN
      | PCIN
      | PCIN+(A+D)
      | PCIN+(A+D)*(A+D)
      | PCIN+(A+D)*(A+D)+C
      | PCIN+(A+D)*(A+D)+C+CARRYIN
      | PCIN+(A+D)*(A+D)+CARRYIN
      | PCIN+(A+D)*(A+D)+P
      | PCIN+(A+D)*(A+D)+P+CARRYIN
      | PCIN+(A+D)*B
      | PCIN+(A+D)*B+C
      | PCIN+(A+D)*B+C+CARRYIN
      | PCIN+(A+D)*B+CARRYIN
      | PCIN+(A+D)*B+P
      | PCIN+(A+D)*B+P+CARRYIN
      | PCIN+(A+D)*BCIN
      | PCIN+(A+D)*BCIN+C
      | PCIN+(A+D)*BCIN+C+CARRYIN
      | PCIN+(A+D)*BCIN+CARRYIN
      | PCIN+(A+D)*BCIN+P
      | PCIN+(A+D)*BCIN+P+CARRYIN
      | PCIN+(A+D)+C
      | PCIN+(A+D)+C+CARRYIN
      | PCIN+(A+D)+CARRYIN
      | PCIN+(A+D)+P
      | PCIN+(A+D)+P+CARRYIN
      | PCIN+(ACIN+D)
      | PCIN+(ACIN+D)*(ACIN+D)
      | PCIN+(ACIN+D)*(ACIN+D)+C
      | PCIN+(ACIN+D)*(ACIN+D)+C+CARRYIN
      | PCIN+(ACIN+D)*(ACIN+D)+CARRYIN
      | PCIN+(ACIN+D)*(ACIN+D)+P
      | PCIN+(ACIN+D)*(ACIN+D)+P+CARRYIN
      | PCIN+(ACIN+D)*B
      | PCIN+(ACIN+D)*B+C
      | PCIN+(ACIN+D)*B+C+CARRYIN
      | PCIN+(ACIN+D)*B+CARRYIN
      | PCIN+(ACIN+D)*B+P
      | PCIN+(ACIN+D)*B+P+CARRYIN
      | PCIN+(ACIN+D)*BCIN
      | PCIN+(ACIN+D)*BCIN+C
      | PCIN+(ACIN+D)*BCIN+C+CARRYIN
      | PCIN+(ACIN+D)*BCIN+CARRYIN
      | PCIN+(ACIN+D)*BCIN+P
      | PCIN+(ACIN+D)*BCIN+P+CARRYIN
      | PCIN+(ACIN+D)+C
      | PCIN+(ACIN+D)+C+CARRYIN
      | PCIN+(ACIN+D)+CARRYIN
      | PCIN+(ACIN+D)+P
      | PCIN+(ACIN+D)+P+CARRYIN
      | PCIN+(D+A)
      | PCIN+(D+A)*(D+A)
      | PCIN+(D+A)*(D+A)+C
      | PCIN+(D+A)*(D+A)+C+CARRYIN
      | PCIN+(D+A)*(D+A)+CARRYIN
      | PCIN+(D+A)*(D+A)+P
      | PCIN+(D+A)*(D+A)+P+CARRYIN
      | PCIN+(D+A)*B
      | PCIN+(D+A)*B+C
      | PCIN+(D+A)*B+C+CARRYIN
      | PCIN+(D+A)*B+CARRYIN
      | PCIN+(D+A)*B+P
      | PCIN+(D+A)*B+P+CARRYIN
      | PCIN+(D+A)*BCIN
      | PCIN+(D+A)*BCIN+C
      | PCIN+(D+A)*BCIN+C+CARRYIN
      | PCIN+(D+A)*BCIN+CARRYIN
      | PCIN+(D+A)*BCIN+P
      | PCIN+(D+A)*BCIN+P+CARRYIN
      | PCIN+(D+A)+C
      | PCIN+(D+A)+C+CARRYIN
      | PCIN+(D+A)+CARRYIN
      | PCIN+(D+A)+P
      | PCIN+(D+A)+P+CARRYIN
      | PCIN+(D+ACIN)
      | PCIN+(D+ACIN)*(D+ACIN)
      | PCIN+(D+ACIN)*(D+ACIN)+C
      | PCIN+(D+ACIN)*(D+ACIN)+C+CARRYIN
      | PCIN+(D+ACIN)*(D+ACIN)+CARRYIN
      | PCIN+(D+ACIN)*(D+ACIN)+P
      | PCIN+(D+ACIN)*(D+ACIN)+P+CARRYIN
      | PCIN+(D+ACIN)*B
      | PCIN+(D+ACIN)*B+C
      | PCIN+(D+ACIN)*B+C+CARRYIN
      | PCIN+(D+ACIN)*B+CARRYIN
      | PCIN+(D+ACIN)*B+P
      | PCIN+(D+ACIN)*B+P+CARRYIN
      | PCIN+(D+ACIN)*BCIN
      | PCIN+(D+ACIN)*BCIN+C
      | PCIN+(D+ACIN)*BCIN+C+CARRYIN
      | PCIN+(D+ACIN)*BCIN+CARRYIN
      | PCIN+(D+ACIN)*BCIN+P
      | PCIN+(D+ACIN)*BCIN+P+CARRYIN
      | PCIN+(D+ACIN)+C
      | PCIN+(D+ACIN)+C+CARRYIN
      | PCIN+(D+ACIN)+CARRYIN
      | PCIN+(D+ACIN)+P
      | PCIN+(D+ACIN)+P+CARRYIN
      | PCIN+(D-A)
      | PCIN+(D-A)*(D-A)
      | PCIN+(D-A)*(D-A)+C
      | PCIN+(D-A)*(D-A)+C+CARRYIN
      | PCIN+(D-A)*(D-A)+CARRYIN
      | PCIN+(D-A)*(D-A)+P
      | PCIN+(D-A)*(D-A)+P+CARRYIN
      | PCIN+(D-A)*B
      | PCIN+(D-A)*B+C
      | PCIN+(D-A)*B+C+CARRYIN
      | PCIN+(D-A)*B+CARRYIN
      | PCIN+(D-A)*B+P
      | PCIN+(D-A)*B+P+CARRYIN
      | PCIN+(D-A)*BCIN
      | PCIN+(D-A)*BCIN+C
      | PCIN+(D-A)*BCIN+C+CARRYIN
      | PCIN+(D-A)*BCIN+CARRYIN
      | PCIN+(D-A)*BCIN+P
      | PCIN+(D-A)*BCIN+P+CARRYIN
      | PCIN+(D-A)+C
      | PCIN+(D-A)+C+CARRYIN
      | PCIN+(D-A)+CARRYIN
      | PCIN+(D-A)+P
      | PCIN+(D-A)+P+CARRYIN
      | PCIN+(D-ACIN)
      | PCIN+(D-ACIN)*(D-ACIN)
      | PCIN+(D-ACIN)*(D-ACIN)+C
      | PCIN+(D-ACIN)*(D-ACIN)+C+CARRYIN
      | PCIN+(D-ACIN)*(D-ACIN)+CARRYIN
      | PCIN+(D-ACIN)*(D-ACIN)+P
      | PCIN+(D-ACIN)*(D-ACIN)+P+CARRYIN
      | PCIN+(D-ACIN)*B
      | PCIN+(D-ACIN)*B+C
      | PCIN+(D-ACIN)*B+C+CARRYIN
      | PCIN+(D-ACIN)*B+CARRYIN
      | PCIN+(D-ACIN)*B+P
      | PCIN+(D-ACIN)*B+P+CARRYIN
      | PCIN+(D-ACIN)*BCIN
      | PCIN+(D-ACIN)*BCIN+C
      | PCIN+(D-ACIN)*BCIN+C+CARRYIN
      | PCIN+(D-ACIN)*BCIN+CARRYIN
      | PCIN+(D-ACIN)*BCIN+P
      | PCIN+(D-ACIN)*BCIN+P+CARRYIN
      | PCIN+(D-ACIN)+C
      | PCIN+(D-ACIN)+C+CARRYIN
      | PCIN+(D-ACIN)+CARRYIN
      | PCIN+(D-ACIN)+P
      | PCIN+(D-ACIN)+P+CARRYIN
      | PCIN+A
      | PCIN+A*A
      | PCIN+A*A+C
      | PCIN+A*A+C+CARRYIN
      | PCIN+A*A+CARRYIN
      | PCIN+A*A+P
      | PCIN+A*A+P+CARRYIN
      | PCIN+A*B
      | PCIN+A*B+C
      | PCIN+A*B+C+CARRYIN
      | PCIN+A*B+CARRYIN
      | PCIN+A*B+P
      | PCIN+A*B+P+CARRYIN
      | PCIN+A*BCIN
      | PCIN+A*BCIN+C
      | PCIN+A*BCIN+C+CARRYIN
      | PCIN+A*BCIN+CARRYIN
      | PCIN+A*BCIN+P
      | PCIN+A*BCIN+P+CARRYIN
      | PCIN+A+C
      | PCIN+A+C+CARRYIN
      | PCIN+A+CARRYIN
      | PCIN+A+P
      | PCIN+A+P+CARRYIN
      | PCIN+ACIN
      | PCIN+ACIN*ACIN
      | PCIN+ACIN*ACIN+C
      | PCIN+ACIN*ACIN+C+CARRYIN
      | PCIN+ACIN*ACIN+CARRYIN
      | PCIN+ACIN*ACIN+P
      | PCIN+ACIN*ACIN+P+CARRYIN
      | PCIN+ACIN*B
      | PCIN+ACIN*B+C
      | PCIN+ACIN*B+C+CARRYIN
      | PCIN+ACIN*B+CARRYIN
      | PCIN+ACIN*B+P
      | PCIN+ACIN*B+P+CARRYIN
      | PCIN+ACIN*BCIN
      | PCIN+ACIN*BCIN+C
      | PCIN+ACIN*BCIN+C+CARRYIN
      | PCIN+ACIN*BCIN+CARRYIN
      | PCIN+ACIN*BCIN+P
      | PCIN+ACIN*BCIN+P+CARRYIN
      | PCIN+ACIN+C
      | PCIN+ACIN+C+CARRYIN
      | PCIN+ACIN+CARRYIN
      | PCIN+ACIN+P
      | PCIN+ACIN+P+CARRYIN
      | PCIN+B*(A+D)
      | PCIN+B*(A+D)+C
      | PCIN+B*(A+D)+C+CARRYIN
      | PCIN+B*(A+D)+CARRYIN
      | PCIN+B*(A+D)+P
      | PCIN+B*(A+D)+P+CARRYIN
      | PCIN+B*(ACIN+D)
      | PCIN+B*(ACIN+D)+C
      | PCIN+B*(ACIN+D)+C+CARRYIN
      | PCIN+B*(ACIN+D)+CARRYIN
      | PCIN+B*(ACIN+D)+P
      | PCIN+B*(ACIN+D)+P+CARRYIN
      | PCIN+B*(D+A)
      | PCIN+B*(D+A)+C
      | PCIN+B*(D+A)+C+CARRYIN
      | PCIN+B*(D+A)+CARRYIN
      | PCIN+B*(D+A)+P
      | PCIN+B*(D+A)+P+CARRYIN
      | PCIN+B*(D+ACIN)
      | PCIN+B*(D+ACIN)+C
      | PCIN+B*(D+ACIN)+C+CARRYIN
      | PCIN+B*(D+ACIN)+CARRYIN
      | PCIN+B*(D+ACIN)+P
      | PCIN+B*(D+ACIN)+P+CARRYIN
      | PCIN+B*(D-A)
      | PCIN+B*(D-A)+C
      | PCIN+B*(D-A)+C+CARRYIN
      | PCIN+B*(D-A)+CARRYIN
      | PCIN+B*(D-A)+P
      | PCIN+B*(D-A)+P+CARRYIN
      | PCIN+B*(D-ACIN)
      | PCIN+B*(D-ACIN)+C
      | PCIN+B*(D-ACIN)+C+CARRYIN
      | PCIN+B*(D-ACIN)+CARRYIN
      | PCIN+B*(D-ACIN)+P
      | PCIN+B*(D-ACIN)+P+CARRYIN
      | PCIN+B*A
      | PCIN+B*A+C
      | PCIN+B*A+C+CARRYIN
      | PCIN+B*A+CARRYIN
      | PCIN+B*A+P
      | PCIN+B*A+P+CARRYIN
      | PCIN+B*ACIN
      | PCIN+B*ACIN+C
      | PCIN+B*ACIN+C+CARRYIN
      | PCIN+B*ACIN+CARRYIN
      | PCIN+B*ACIN+P
      | PCIN+B*ACIN+P+CARRYIN
      | PCIN+B*D
      | PCIN+B*D+C
      | PCIN+B*D+C+CARRYIN
      | PCIN+B*D+CARRYIN
      | PCIN+B*D+P
      | PCIN+B*D+P+CARRYIN
      | PCIN+BCIN*(A+D)
      | PCIN+BCIN*(A+D)+C
      | PCIN+BCIN*(A+D)+C+CARRYIN
      | PCIN+BCIN*(A+D)+CARRYIN
      | PCIN+BCIN*(A+D)+P
      | PCIN+BCIN*(A+D)+P+CARRYIN
      | PCIN+BCIN*(ACIN+D)
      | PCIN+BCIN*(ACIN+D)+C
      | PCIN+BCIN*(ACIN+D)+C+CARRYIN
      | PCIN+BCIN*(ACIN+D)+CARRYIN
      | PCIN+BCIN*(ACIN+D)+P
      | PCIN+BCIN*(ACIN+D)+P+CARRYIN
      | PCIN+BCIN*(D+A)
      | PCIN+BCIN*(D+A)+C
      | PCIN+BCIN*(D+A)+C+CARRYIN
      | PCIN+BCIN*(D+A)+CARRYIN
      | PCIN+BCIN*(D+A)+P
      | PCIN+BCIN*(D+A)+P+CARRYIN
      | PCIN+BCIN*(D+ACIN)
      | PCIN+BCIN*(D+ACIN)+C
      | PCIN+BCIN*(D+ACIN)+C+CARRYIN
      | PCIN+BCIN*(D+ACIN)+CARRYIN
      | PCIN+BCIN*(D+ACIN)+P
      | PCIN+BCIN*(D+ACIN)+P+CARRYIN
      | PCIN+BCIN*(D-A)
      | PCIN+BCIN*(D-A)+C
      | PCIN+BCIN*(D-A)+C+CARRYIN
      | PCIN+BCIN*(D-A)+CARRYIN
      | PCIN+BCIN*(D-A)+P
      | PCIN+BCIN*(D-A)+P+CARRYIN
      | PCIN+BCIN*(D-ACIN)
      | PCIN+BCIN*(D-ACIN)+C
      | PCIN+BCIN*(D-ACIN)+C+CARRYIN
      | PCIN+BCIN*(D-ACIN)+CARRYIN
      | PCIN+BCIN*(D-ACIN)+P
      | PCIN+BCIN*(D-ACIN)+P+CARRYIN
      | PCIN+BCIN*A
      | PCIN+BCIN*A+C
      | PCIN+BCIN*A+C+CARRYIN
      | PCIN+BCIN*A+CARRYIN
      | PCIN+BCIN*A+P
      | PCIN+BCIN*A+P+CARRYIN
      | PCIN+BCIN*ACIN
      | PCIN+BCIN*ACIN+C
      | PCIN+BCIN*ACIN+C+CARRYIN
      | PCIN+BCIN*ACIN+CARRYIN
      | PCIN+BCIN*ACIN+P
      | PCIN+BCIN*ACIN+P+CARRYIN
      | PCIN+BCIN*D
      | PCIN+BCIN*D+C
      | PCIN+BCIN*D+C+CARRYIN
      | PCIN+BCIN*D+CARRYIN
      | PCIN+BCIN*D+P
      | PCIN+BCIN*D+P+CARRYIN
      | PCIN+C
      | PCIN+C+(A+D)
      | PCIN+C+(A+D)*(A+D)
      | PCIN+C+(A+D)*(A+D)+CARRYIN
      | PCIN+C+(A+D)*B
      | PCIN+C+(A+D)*B+CARRYIN
      | PCIN+C+(A+D)*BCIN
      | PCIN+C+(A+D)*BCIN+CARRYIN
      | PCIN+C+(A+D)+CARRYIN
      | PCIN+C+(ACIN+D)
      | PCIN+C+(ACIN+D)*(ACIN+D)
      | PCIN+C+(ACIN+D)*(ACIN+D)+CARRYIN
      | PCIN+C+(ACIN+D)*B
      | PCIN+C+(ACIN+D)*B+CARRYIN
      | PCIN+C+(ACIN+D)*BCIN
      | PCIN+C+(ACIN+D)*BCIN+CARRYIN
      | PCIN+C+(ACIN+D)+CARRYIN
      | PCIN+C+(D+A)
      | PCIN+C+(D+A)*(D+A)
      | PCIN+C+(D+A)*(D+A)+CARRYIN
      | PCIN+C+(D+A)*B
      | PCIN+C+(D+A)*B+CARRYIN
      | PCIN+C+(D+A)*BCIN
      | PCIN+C+(D+A)*BCIN+CARRYIN
      | PCIN+C+(D+A)+CARRYIN
      | PCIN+C+(D+ACIN)
      | PCIN+C+(D+ACIN)*(D+ACIN)
      | PCIN+C+(D+ACIN)*(D+ACIN)+CARRYIN
      | PCIN+C+(D+ACIN)*B
      | PCIN+C+(D+ACIN)*B+CARRYIN
      | PCIN+C+(D+ACIN)*BCIN
      | PCIN+C+(D+ACIN)*BCIN+CARRYIN
      | PCIN+C+(D+ACIN)+CARRYIN
      | PCIN+C+(D-A)
      | PCIN+C+(D-A)*(D-A)
      | PCIN+C+(D-A)*(D-A)+CARRYIN
      | PCIN+C+(D-A)*B
      | PCIN+C+(D-A)*B+CARRYIN
      | PCIN+C+(D-A)*BCIN
      | PCIN+C+(D-A)*BCIN+CARRYIN
      | PCIN+C+(D-A)+CARRYIN
      | PCIN+C+(D-ACIN)
      | PCIN+C+(D-ACIN)*(D-ACIN)
      | PCIN+C+(D-ACIN)*(D-ACIN)+CARRYIN
      | PCIN+C+(D-ACIN)*B
      | PCIN+C+(D-ACIN)*B+CARRYIN
      | PCIN+C+(D-ACIN)*BCIN
      | PCIN+C+(D-ACIN)*BCIN+CARRYIN
      | PCIN+C+(D-ACIN)+CARRYIN
      | PCIN+C+A
      | PCIN+C+A*A
      | PCIN+C+A*A+CARRYIN
      | PCIN+C+A*B
      | PCIN+C+A*B+CARRYIN